simulink model "vegas01_4tap_1024ch_250mhz_r1"  (Simulink 7.6)
settings: Solver=ode45  StartTime=0.0  StopTime=128000  RelTol=1e-3
undefined symbols (not defined in the file): ROACH
libraries: xbsIndex_r4 x12350, monroe_library x84, xbsReplacements_r4 x36, xps_library x30, casper_library_misc x24, dspsigops x16
(part 7 of 103)

== system vegas01_4tap_1024ch_250mhz_r1/pfb_fir_real1/coeff_gen_3_4 ==
  sync : Inport
  Counter0 : xbsIndex_r4/Counter  cnt_type=Free Running  cnt_to=Inf  operation=Up  start_count=0  cnt_by_val=1  arith_type=Unsigned  n_bits=8  bin_pt=0  load_pin=off  rst=on  en=off  explicit_period=on  period=1  dbl_ovrd=off  use_behavioral_HDL=off  implementation=Fabric  block_type=counter
  Counter1 : xbsIndex_r4/Counter  cnt_type=Free Running  cnt_to=Inf  operation=Down  start_count=255  cnt_by_val=1  arith_type=Unsigned  n_bits=8  bin_pt=0  load_pin=off  rst=on  en=off  explicit_period=on  period=1  dbl_ovrd=off  use_behavioral_HDL=off  implementation=Fabric  block_type=counter
  coeff_ram_1 : xbsIndex_r4/Dual Port RAM  depth=256  initVector=[-5.86373825914179e-05 -0.000215456147790523 -0.000372998021380911 -0.000531345655026345 -0.000690582222902466 -0.000850791372601726 -0.00101205717553319 -0.00117446407683278 -0.00133809684480029 -0.0015030405198794 -0.00166938036319721 -0.00183720180467995 -0.00200659039076204 -0.0021776317317052 -0.00235041144854523 -0.00252501511968397 -0.00270152822714364 -0.00288003610250207 -0.00306062387252614 -0.00324337640452213 -0.00342837825142082 -0.00361571359661625 -0.00380546619857627 -0.00399771933524413 -0.00419255574824945 -0.00439005758694802 -0.00459030635230934 -0.00479338284067104 -0.00499936708737985 -0.00520833831033822 -0.00542037485347644 -0.00563555413016958 -0.00585395256661942 -0.0060756455452207 -0.00630070734793194 -0.00652921109967067 -0.00676122871175293 -0.00699683082539746 -0.00723608675531428 -0.00747906443339831 -0.00772583035254768 -0.00797644951062753 -0.00823098535459909 -0.00848949972483472 -0.00875205279963877 -0.00901870303999501 -0.00928950713456065 -0.00956451994492721 -0.0098437944511688 -0.0101273816976976 -0.0104153307394476 -0.0107076885884055 -0.0110045001605102 -0.0113058082229405 -0.0116116533418109 -0.0119220738302957 -0.0122371056972012 -0.0125567825960062 -0.0128811357743903 -0.0132101940242697 -0.0135439836323603 -0.0138825283312874 -0.0142258492512617 -0.0145739648723409 -0.0149268909772952 -0.0152846406050976 -0.0156472240050563 -0.0160146485916082 -0.0163869188997935 -0.0167640365414281 -0.0171460001619934 -0.0175328053982617 -0.0179244448366743 -0.0183209079724907 -0.0187221811697278 -0.0191282476219039 -0.0195390873136075 -0.0199546769829069 -0.0203749900846166 -0.0207999967544396 -0.0212296637739995 -0.0216639545367806 -0.0221028290149906 -0.0225462437273637 -0.0229941517079172 -0.0234465024756791 -0.0239032420054004 -0.0243643126992679 -0.0248296533596316 -0.0252991991627612 -0.0257728816336456 -0.0262506286218495 -0.0267323642784407 -0.0272180090340002 -0.0277074795777305 -0.0282006888376717 -0.0286975459620406 -0.0291979563017022 -0.0297018213937885 -0.0302090389464727 -0.0307195028249126 -0.0312331030383722 -0.0317497257285335 -0.0322692531590073 -0.0327915637060538 -0.0333165318505221 -0.033844028171018 -0.0343739193383085 -0.0349060681109713 -0.0354403333322995 -0.0359765699284654 -0.0365146289079547 -0.0370543573622759 -0.0375955984679522 -0.0381381914898021 -0.038681971785515 -0.0392267708115271 -0.0397724161302025 -0.0403187314183254 -0.0408655364769064 -0.0414126472423082 -0.0419598757986934 -0.0425070303917987 -0.0430539154440375 -0.043600331570934 -0.0441460755988906 -0.0446909405842904 -0.0452347158339363 -0.0457771869268272 -0.0463181357372728 -0.0468573404593456 -0.047394575632671 -0.047929612169555 -0.0484622173834472 -0.0489921550187384 -0.0495191852818918 -0.0500430648739029 -0.0505635470240889 -0.0510803815252008 -0.051593314769858 -0.0521020897882994 -0.0526064462874469 -0.0531061206912774 -0.0536008461824974 -0.0540903527455154 -0.0545743672107048 -0.0550526132999523 -0.0555248116734847 -0.0559906799779658 -0.056449932895858 -0.0569022821960385 -0.0573474367856626 -0.0577851027632654 -0.058214983473093 -0.0586367795606527 -0.0590501890294728 -0.0594549072990618 -0.0598506272640558 -0.0602370393545433 -0.0606138315975561 -0.0609806896797129 -0.0613372970110058 -0.0616833347897144 -0.0620184820684369 -0.0623424158212223 -0.0626548110117918 -0.062955340662835 -0.0632436759263647 -0.0635194861551178 -0.0637824389749851 -0.0640322003584556 -0.0642684346990587 -0.064490804886788 -0.0646989723844908 -0.0648925973052045 -0.0650713384904255 -0.0652348535892895 -0.0653827991386482 -0.0655148306440217 -0.0656306026614101 -0.0657297688799434 -0.0658119822053517 -0.0658768948442361 -0.0659241583891191 -0.0659534239042566 -0.0659643420121883 -0.0659565629810087 -0.065929736812335 -0.0658835133299523 -0.0658175422691142 -0.0657314733664758 -0.0656249564506396 -0.065497641533289 -0.0653491789008882 -0.0651792192069268 -0.0649874135646831 -0.0647734136404861 -0.0645368717474501 ...(+1155 chars)]  distributed_mem=Block RAM  init_a=0  init_b=0  rst_a=off  rst_b=off  en_a=off  en_b=off  latency=3  write_mode_A=No Read On Write  write_mode_B=No Read On Write  dbl_ovrd=off  optimize=Area  use_rpm=on  block_type=dpram
  coeff_ram_2 : xbsIndex_r4/Dual Port RAM  depth=256  initVector=[0.000793855568781102 0.00293734991211368 0.00511955769539444 0.00734060096668454 0.00960059539061112 0.0118996501782322 0.0142378680181029 0.016615345008565 0.0190321705912827 0.0214884274860464 0.0239841916268662 0.0265195320993763 0.0290945110795724 0.0317091837739016 0.0343635983607266 0.0370577959331841 0.0397918104434568 0.04256566864848 0.0453793900570998 0.0482329868787049 0.051126463973348 0.0540598188033769 0.0570330413865915 0.0600461142509466 0.0630990123908156 0.066191703224833 0.0693241465553323 0.072496294529395 0.0757080916015266 0.0789594744979745 0.0822503721827049 0.0855807058250493 0.0889503887690387 0.0923593265044371 0.095807416639488 0.0992945488753877 0.102820604982496 0.1063854587783 0.109988976107135 0.113631014821687 0.117311424766273 0.121030047761919 0.124786717593239 0.128581259997137 0.132413492653321 0.136283225176654 0.140190259111347 0.144134387926999 0.148115397016481 0.152133063695701 0.156187157205215 0.16027743871373 0.164403661323472 0.168565570077445 0.172762901968574 0.176995385950742 0.18126274295172 0.185564685887996 0.189900919681506 0.19427114127826 0.198675039668881 0.203112295911043 0.207582583153816 0.21208556666391 0.216620903853836 0.221188244311956 0.225787229834444 0.230417494459145 0.235078664501327 0.239770358591334 0.244492187714121 0.249243755250686 0.254024657021376 0.258834481331072 0.263672809016247 0.268539213493888 0.273433260812281 0.278354509703641 0.283302511638591 0.28827681088248 0.293276944553524 0.29830244268277 0.303352828275865 0.308427617376632 0.31352631913243 0.318648435861293 0.323793463120839 0.328960889778932 0.334150198086085 0.339360863749602 0.344592356009424 0.349844137715687 0.355115665407971 0.360406389396214 0.365715753843293 0.371043196849245 0.376388150537122 0.381750041140444 0.387128289092261 0.392522309115784 0.397931510316576 0.403355296276285 0.408793065147898 0.414244209752503 0.419708117677526 0.42518417137644 0.430671748269917 0.436170220848394 0.441678956776052 0.447197318996171 0.452724665837839 0.458260351124004 0.463803724280834 0.469354130448373 0.474910910592456 0.480473401617876 0.486040936482762 0.491612844314159 0.497188450524771 0.502767076930856 0.508348041871229 0.513930660327374 0.519514244044604 0.525098101654283 0.530681538797043 0.536263858246998 0.541844360036917 0.547422341584325 0.552997097818512 0.558567921308413 0.564134102391344 0.569694929302543 0.575249688305515 0.580797663823129 0.586338138569444 0.591870393682245 0.597393708856244 0.602907362476923 0.608410631754986 0.613902792861399 0.61938312106297 0.624850890858456 0.630305376115151 0.635745850205934 0.641171586146744 0.646581856734442 0.651975934685042 0.657353092772267 0.662712603966406 0.668053741573435 0.673375779374372 0.678677991764833 0.683959653894763 0.689220041808292 0.694458432583704 0.699674104473478 0.704866337044359 0.710034411317448 0.715177609908251 0.72029521716668 0.725386519316956 0.730450804597387 0.735487363399991 0.740495488409925 0.745474474744689 0.750423620093078 0.755342224853842 0.760229592274024 0.76508502858694 0.769907843149779 0.774697348580773 0.779452860895924 0.784173699645239 0.788859188048447 0.793508653130172 0.79812142585452 0.802696841259047 0.807234238588094 0.811732961425428 0.816192357826184 0.820611780448065 0.824990586681763 0.829328138780581 0.83362380398922 0.837876954671699 0.842086968438377 0.846253228272049 0.850375122653088 0.854452045683591 0.858483397210515 0.862468582947766 0.866407014597203 0.870298109968547 0.874141293098149 0.877935994366595 0.88168165061512 0.885377705260805 0.889023608410519 0.892618816973592 0.896162794773185 0.899655012656325 0.90309494860259 0.906482087831407 0.909815922907943 0.913095953847561 0.916321688218814 0.919492641244963 0.922608335903972 0.925668303026978 0.928672081395204 0.931619217835282 0.934509267312983 0.937341793025309 0.940116366490941 0.942832567639016 0.945489984896204 0.948088215272075 0.950626864442732 0.953105546832681 0.955523885694932 0.9578815131893 ...(+626 chars)]  distributed_mem=Block RAM  init_a=0  init_b=0  rst_a=off  rst_b=off  en_a=off  en_b=off  latency=3  write_mode_A=No Read On Write  write_mode_B=No Read On Write  dbl_ovrd=off  optimize=Area  use_rpm=on  block_type=dpram
  coeff_ram_3 : xbsIndex_r4/Dual Port RAM  depth=256  initVector=[0.999994812497531 0.999934649315113 0.999806974692208 0.999611808340819 0.999349180372279 0.999019131290932 0.998621711985642 0.998156983719109 0.99762501811503 0.997025897143069 0.996359713101675 0.995626568598733 0.994826576530044 0.993959860055675 0.993026552574138 0.992026797694442 0.990960749206007 0.989828571046454 0.988630437267265 0.987366531997347 0.986037049404487 0.984642193654713 0.983182178869577 0.981657229081366 0.980067578186247 0.978413469895374 0.976695157683941 0.974912904738229 0.973066983900625 0.971157677612657 0.96918527785603 0.967150086091704 0.965052413197015 0.962892579400849 0.9606709142169 0.95838775637502 0.956043453750677 0.953638363292534 0.951172850948186 0.948647291588047 0.946062068927424 0.94341757544679 0.940714212310279 0.937952389282417 0.935132524643115 0.932255045100944 0.929320385704707 0.926328989753344 0.923281308704171 0.920177802079496 0.917018937371619 0.913805189946252 0.910537042944371 0.907214987182531 0.903839521051669 0.90041115041441 0.896930388500917 0.893397755803297 0.889813779968586 0.886178995690362 0.88249394459898 0.878759175150481 0.87497524251419 0.871142708459034 0.867262141238604 0.863334115474993 0.85935921204144 0.855338017943796 0.851271126200856 0.847159135723579 0.843002651193223 0.83880228293843 0.834558646811279 0.83027236406236 0.825944061214867 0.821574369937774 0.817163926918096 0.81271337373228 0.808223356716763 0.803694526837708 0.799127539559968 0.7945230547153 0.789881736369862 0.785204252691029 0.780491275813551 0.775743481705095 0.770961550031191 0.766146164019636 0.761298010324352 0.756417778888776 0.751506162808768 0.74656385819511 0.741591564035602 0.736589982056795 0.731559816585398 0.726501774409391 0.721416564638871 0.716304898566669 0.711167489528765 0.706005052764548 0.700818305276932 0.695607965692387 0.690374754120884 0.685119392015829 0.679842602033979 0.674545107895397 0.669227634243474 0.663890906505042 0.658535650750622 0.653162593554832 0.647772461856991 0.642365982821949 0.636943883701178 0.631506891694151 0.626055733810047 0.620591136729813 0.61511382666861 0.609624529238677 0.604123969312646 0.598612870887343 0.593091956948089 0.587561949333563 0.582023568601217 0.576477533893316 0.570924562803598 0.56536537124461 0.559800673315729 0.554231181171917 0.548657604893226 0.543080652355085 0.537501029099403 0.53191943820651 0.526336580167975 0.520753152760311 0.515169850919615 0.50958736661716 0.504006388735964 0.498427602948374 0.492851691594681 0.487279333562795 0.481711204169014 0.476147975039901 0.470590313995303 0.465038884932535 0.459494347711752 0.453957358042535 0.448428567371718 0.442908622772472 0.437398166834681 0.431897837556619 0.426408268237969 0.420930087374181 0.415463918552222 0.410010380347709 0.404570086223473 0.39914364442955 0.393731657904643 0.388334724179058 0.382953435279135 0.377588377633211 0.372240131979102 0.366909273273159 0.361596370600881 0.35630198708913 0.351026679819948 0.345770999746003 0.340535491607668 0.335320693851764 0.330127138551971 0.324955351330922 0.319805851284001 0.314679150904859 0.309575756012646 0.304496165680994 0.299440872168748 0.294410360852464 0.28940511016068 0.284425591509976 0.279472269242834 0.274545600567304 0.269646035498482 0.264774016801823 0.259929979938285 0.255114353011316 0.250327556715688 0.245570004288195 0.240842101460215 0.236144246412134 0.231476829729653 0.226840234361979 0.222234835581892 0.21766100094771 0.213119090267143 0.208609455563046 0.20413244104107 0.199688383059216 0.195277610099287 0.19090044274025 0.186557193633502 0.182248167480034 0.177973661009513 0.173733962961252 0.169529354067092 0.165360107036185 0.161226486541674 0.15712874920926 0.153067143607678 0.149041910241047 0.14505328154311 0.141101481873355 0.137186727515006 0.133309226674887 0.129469179485146 0.125666778006837 0.121902206235346 0.118175640107666 0.114487247511497 0.110837188296181 0.107225614285441 0.103652669291933 0.100118489133601 0.0966232016518021 0.0931669267312255 ...(+627 chars)]  distributed_mem=Block RAM  init_a=0  init_b=0  rst_a=off  rst_b=off  en_a=off  en_b=off  latency=3  write_mode_A=No Read On Write  write_mode_B=No Read On Write  dbl_ovrd=off  optimize=Area  use_rpm=on  block_type=dpram
  coeff_ram_4 : xbsIndex_r4/Dual Port RAM  depth=256  initVector=[-0.000787920351828344 -0.00286260996943633 -0.00489896520129326 -0.00689713161888919 -0.00885726082449819 -0.0107795103745431 -0.0126640437019187 -0.0145110300372983 -0.0163206443294465 -0.0180930671645657 -0.0198284846846987 -0.0215270885052133 -0.0231890756313952 -0.0248146483741729 -0.0264040142650019 -0.0279573859699323 -0.0294749812028871 -0.0309570226381763 -0.0324037378222731 -0.0338153590848793 -0.0351921234493043 -0.036534272542187 -0.0378420525025839 -0.0391157138904531 -0.0403555115945585 -0.0415617047398231 -0.042734556594156 -0.0438743344747823 -0.0449813096541012 -0.0460557572651 -0.0470979562063509 -0.0481081890466181 -0.0490867419291014 -0.0500339044753439 -0.0509499696888316 -0.0518352338583101 -0.0526899964608479 -0.0535145600646716 -0.0543092302318 -0.0550743154205058 -0.0558101268876299 -0.0565169785907762 -0.0571951870904141 -0.0578450714519141 -0.0584669531475448 -0.0590611559584568 -0.0596280058766808 -0.0601678310071659 -0.0606809614698843 -0.0611677293020293 -0.0616284683603321 -0.0620635142235239 -0.0624732040949686 -0.0628578767054923 -0.0632178722164352 -0.0635535321229509 -0.063865199157579 -0.0641532171941154 -0.0644179311518061 -0.0646596868998882 -0.0648788311625045 -0.0650757114240131 -0.0652506758347198 -0.0654040731170541 -0.065536252472215 -0.0656475634873088 -0.0657383560430019 -0.0658089802217137 -0.0658597862163704 -0.0658911242397428 -0.0659033444343921 -0.0658967967832431 -0.0658718310208095 -0.0658287965450905 -0.0657680423301619 -0.0656899168394816 -0.0655947679399311 -0.0654829428166129 -0.0653547878884241 -0.0652106487244269 -0.0650508699610347 -0.0648757952200333 -0.0646857670274563 -0.0644811267333347 -0.0642622144323359 -0.0640293688853139 -0.0637829274417857 -0.0635232259633528 -0.0632505987480838 -0.0629653784558762 -0.0626678960348121 -0.0623584806485253 -0.0620374596045948 -0.0617051582839797 -0.0613619000715118 -0.0610080062874585 -0.0606437961201723 -0.0602695865598394 -0.0598856923333414 -0.0594924258402432 -0.0590900970899198 -0.0586790136398347 -0.0582594805349814 -0.0578318002484996 -0.0573962726234782 -0.0569531948159544 -0.0565028612391215 -0.0560455635087529 -0.0555815903898541 -0.0551112277445508 -0.0546347584812225 -0.0541524625048896 -0.0536646166688625 -0.0531714947276601 -0.0526733672912054 -0.0521705017803039 -0.051663162383413 -0.051151610014707 -0.0506361022734436 -0.0501168934046382 -0.0495942342610489 -0.0490683722664788 -0.0485395513803979 -0.0480080120638888 -0.0474739912469198 -0.0469377222969473 -0.0463994349888512 -0.0458593554762035 -0.0453177062638732 -0.0447747061819681 -0.0442305703611143 -0.0436855102090731 -0.0431397333886967 -0.0425934437972209 -0.0420468415468947 -0.0415001229469451 -0.0409534804868759 -0.040407102821098 -0.0398611747548894 -0.0393158772316811 -0.0387713873216666 -0.0382278782117312 -0.0376855191966968 -0.0371444756718779 -0.0366049091269439 -0.0360669771410839 -0.035530833379466 -0.0349966275909875 -0.0344645056073088 -0.0339346093431641 -0.0334070767979427 -0.0328820420585328 -0.0323596353034207 -0.0318399828080369 -0.0313232069513411 -0.0308094262236367 -0.0302987552356068 -0.0297913047285612 -0.0292871815858849 -0.0287864888456782 -0.0282893257145784 -0.0277957875827514 -0.0273059660400422 -0.0268199488932742 -0.0263378201846839 -0.0258596602114802 -0.0253855455465156 -0.024915549060056 -0.0244497399426378 -0.0239881837289974 -0.0235309423230611 -0.0230780740239801 -0.0226296335531983 -0.0221856720825369 -0.0217462372632829 -0.0213113732562652 -0.0208811207629043 -0.0204555170572198 -0.02003459601878 -0.0196183881665788 -0.019206920693822 -0.0188002175036087 -0.0183982992454906 -0.0180011833528919 -0.0176088840813737 -0.0172214125477258 -0.0168387767698682 -0.0164609817075447 -0.0160880293037919 -0.0157199185271648 -0.015356645414701 -0.0149982031156059 -0.0146445819356409 -0.0142957693821939 -0.013951750210017 -0.0136125064676089 -0.0132780175442264 -0.012948260217503 -0.012623208701658 -0.0123028346962748 ...(+1170 chars)]  distributed_mem=Block RAM  init_a=0  init_b=0  rst_a=off  rst_b=off  en_a=off  en_b=off  latency=3  write_mode_A=No Read On Write  write_mode_B=No Read On Write  dbl_ovrd=off  optimize=Area  use_rpm=on  block_type=dpram
  const_din : xbsIndex_r4/Constant  arith_type=Signed (2's comp)  const=0  n_bits=18  bin_pt=17  explicit_period=on  period=1  equ=P=C  opselect=C  inp2=PCIN>>17  opr=+  inp1=P  carry=CIN  dbl_ovrd=off  block_type=constant
  const_we : xbsIndex_r4/Constant  arith_type=Boolean  const=0  n_bits=16  bin_pt=14  explicit_period=on  period=1  equ=P=C  opselect=C  inp2=PCIN>>17  opr=+  inp1=P  carry=CIN  dbl_ovrd=off  block_type=constant
  cram_lower : Subsystem
  cram_upper : Subsystem
  delay_a_addr1 : xbsIndex_r4/Delay  en=off  latency=2  dbl_ovrd=off  reg_retiming=off  block_type=delay
  delay_a_addr2 : xbsIndex_r4/Delay  en=off  latency=1  dbl_ovrd=off  reg_retiming=off  block_type=delay
  delay_b_addr3 : xbsIndex_r4/Delay  en=off  latency=1  dbl_ovrd=off  reg_retiming=off  block_type=delay
  delay_b_addr4 : xbsIndex_r4/Delay  en=off  latency=2  dbl_ovrd=off  reg_retiming=off  block_type=delay
  delay_sync : xbsIndex_r4/Delay  en=off  latency=3  dbl_ovrd=off  reg_retiming=off  block_type=delay
  sync_out : Outport
  coeff : Outport  Port=2
  coeff_rev : Outport  Port=3
  wires:
  sync/1 -> Counter1/1, Counter0/1, delay_sync/1
  Counter0/1 -> coeff_ram_4/1, coeff_ram_3/1, delay_a_addr2/1, delay_a_addr1/1
  const_din/1 -> coeff_ram_4/5, coeff_ram_4/2, coeff_ram_3/5, coeff_ram_3/2, coeff_ram_2/5, coeff_ram_2/2, coeff_ram_1/5, coeff_ram_1/2
  const_we/1 -> coeff_ram_4/6, coeff_ram_4/3, coeff_ram_3/6, coeff_ram_3/3, coeff_ram_2/6, coeff_ram_2/3, coeff_ram_1/6, coeff_ram_1/3
  Counter1/1 -> delay_b_addr4/1, delay_b_addr3/1, coeff_ram_2/4, coeff_ram_1/4
  cram_lower/1(out) -> coeff_rev/1
  cram_upper/1(out) -> coeff/1
  coeff_ram_1/2 -> cram_lower/4(in3)
  coeff_ram_2/2 -> cram_lower/3(in2)
  coeff_ram_3/2 -> cram_lower/2(in1)
  coeff_ram_4/2 -> cram_lower/1(in0)
  coeff_ram_4/1 -> cram_upper/4(in3)
  coeff_ram_3/1 -> cram_upper/3(in2)
  coeff_ram_2/1 -> cram_upper/2(in1)
  coeff_ram_1/1 -> cram_upper/1(in0)
  delay_b_addr4/1 -> coeff_ram_4/4
  delay_b_addr3/1 -> coeff_ram_3/4
  delay_a_addr2/1 -> coeff_ram_2/1
  delay_a_addr1/1 -> coeff_ram_1/1
  delay_sync/1 -> sync_out/1

== system vegas01_4tap_1024ch_250mhz_r1/pfb_fir_real1/multi_delay_bram_fast_0 ==
  in0 : Inport
  in1 : Inport  Port=2
  in2 : Inport  Port=3
  in3 : Inport  Port=4
  in4 : Inport  Port=5
  in5 : Inport  Port=6
  Concat2_1 : xbsIndex_r4/Concat  num_inputs=2  dbl_ovrd=off  block_type=concat
  cram : Subsystem
  cram_all : Subsystem
  double_delay_bram_fast1 : monroe_library/double_delay_bram_fast  SystemSampleTime=-1  delay_len=256  bram_latency=2  register_counter=1
  double_delay_bram_fast2 : monroe_library/delay_bram_fast  SystemSampleTime=-1  delay_len=256  bram_latency=2  memory_type=Block RAM  register_counter=1
  sliceA_1 : xbsIndex_r4/Slice  nbits=18  boolean_output=off  mode=Upper Bit Location + Width  bit1=-0  base1=MSB of Input  bit0=-0  base0=MSB of Input  dbl_ovrd=off  block_type=slice
  sliceB_1 : xbsIndex_r4/Slice  nbits=18  boolean_output=off  mode=Upper Bit Location + Width  bit1=-18  base1=MSB of Input  bit0=-18  base0=MSB of Input  dbl_ovrd=off  block_type=slice
  slice_last : xbsIndex_r4/Slice  nbits=12  boolean_output=off  mode=Lower Bit Location + Width  bit1=0  base1=LSB of Input  bit0=0  base0=LSB of Input  dbl_ovrd=off  block_type=slice
  uncram : Subsystem
  out0 : Outport
  out1 : Outport  Port=2
  out2 : Outport  Port=3
  out3 : Outport  Port=4
  out4 : Outport  Port=5
  out5 : Outport  Port=6
  wires:
  cram/1(out) -> slice_last/1, sliceB_1/1, sliceA_1/1
  uncram/6(out5) -> out5/1
  uncram/5(out4) -> out4/1
  uncram/4(out3) -> out3/1
  uncram/3(out2) -> out2/1
  uncram/2(out1) -> out1/1
  uncram/1(out0) -> out0/1
  cram_all/1(out) -> uncram/1(in)
  double_delay_bram_fast2/1 -> cram_all/2(in1)
  Concat2_1/1 -> cram_all/1(in0)
  slice_last/1 -> double_delay_bram_fast2/1
  double_delay_bram_fast1/2 -> Concat2_1/2
  double_delay_bram_fast1/1 -> Concat2_1/1
  sliceB_1/1 -> double_delay_bram_fast1/2
  sliceA_1/1 -> double_delay_bram_fast1/1
  in5/1 -> cram/6(in5)
  in4/1 -> cram/5(in4)
  in3/1 -> cram/4(in3)
  in2/1 -> cram/3(in2)
  in1/1 -> cram/2(in1)
  in0/1 -> cram/1(in0)

== system vegas01_4tap_1024ch_250mhz_r1/pfb_fir_real1/multi_delay_bram_fast_1 ==
  in0 : Inport
  in1 : Inport  Port=2
  in2 : Inport  Port=3
  in3 : Inport  Port=4
  in4 : Inport  Port=5
  in5 : Inport  Port=6
  Concat2_1 : xbsIndex_r4/Concat  num_inputs=2  dbl_ovrd=off  block_type=concat
  cram : Subsystem
  cram_all : Subsystem
  double_delay_bram_fast1 : monroe_library/double_delay_bram_fast  SystemSampleTime=-1  delay_len=256  bram_latency=2  register_counter=1
  double_delay_bram_fast2 : monroe_library/delay_bram_fast  SystemSampleTime=-1  delay_len=256  bram_latency=2  memory_type=Block RAM  register_counter=1
  sliceA_1 : xbsIndex_r4/Slice  nbits=18  boolean_output=off  mode=Upper Bit Location + Width  bit1=-0  base1=MSB of Input  bit0=-0  base0=MSB of Input  dbl_ovrd=off  block_type=slice
  sliceB_1 : xbsIndex_r4/Slice  nbits=18  boolean_output=off  mode=Upper Bit Location + Width  bit1=-18  base1=MSB of Input  bit0=-18  base0=MSB of Input  dbl_ovrd=off  block_type=slice
  slice_last : xbsIndex_r4/Slice  nbits=12  boolean_output=off  mode=Lower Bit Location + Width  bit1=0  base1=LSB of Input  bit0=0  base0=LSB of Input  dbl_ovrd=off  block_type=slice
  uncram : Subsystem
  out0 : Outport
  out1 : Outport  Port=2
  out2 : Outport  Port=3
  out3 : Outport  Port=4
  out4 : Outport  Port=5
  out5 : Outport  Port=6
  wires:
  cram/1(out) -> slice_last/1, sliceB_1/1, sliceA_1/1
  uncram/6(out5) -> out5/1
  uncram/5(out4) -> out4/1
  uncram/4(out3) -> out3/1
  uncram/3(out2) -> out2/1
  uncram/2(out1) -> out1/1
  uncram/1(out0) -> out0/1
  cram_all/1(out) -> uncram/1(in)
  double_delay_bram_fast2/1 -> cram_all/2(in1)
  Concat2_1/1 -> cram_all/1(in0)
  slice_last/1 -> double_delay_bram_fast2/1
  double_delay_bram_fast1/2 -> Concat2_1/2
  double_delay_bram_fast1/1 -> Concat2_1/1
  sliceB_1/1 -> double_delay_bram_fast1/2
  sliceA_1/1 -> double_delay_bram_fast1/1
  in5/1 -> cram/6(in5)
  in4/1 -> cram/5(in4)
  in3/1 -> cram/4(in3)
  in2/1 -> cram/3(in2)
  in1/1 -> cram/2(in1)
  in0/1 -> cram/1(in0)

== system vegas01_4tap_1024ch_250mhz_r1/pfb_fir_real1/multi_delay_bram_fast_2 ==
  in0 : Inport
  in1 : Inport  Port=2
  in2 : Inport  Port=3
  in3 : Inport  Port=4
  in4 : Inport  Port=5
  in5 : Inport  Port=6
  Concat2_1 : xbsIndex_r4/Concat  num_inputs=2  dbl_ovrd=off  block_type=concat
  cram : Subsystem
  cram_all : Subsystem
  double_delay_bram_fast1 : monroe_library/double_delay_bram_fast  SystemSampleTime=-1  delay_len=256  bram_latency=2  register_counter=1
  double_delay_bram_fast2 : monroe_library/delay_bram_fast  SystemSampleTime=-1  delay_len=256  bram_latency=2  memory_type=Block RAM  register_counter=1
  sliceA_1 : xbsIndex_r4/Slice  nbits=18  boolean_output=off  mode=Upper Bit Location + Width  bit1=-0  base1=MSB of Input  bit0=-0  base0=MSB of Input  dbl_ovrd=off  block_type=slice
  sliceB_1 : xbsIndex_r4/Slice  nbits=18  boolean_output=off  mode=Upper Bit Location + Width  bit1=-18  base1=MSB of Input  bit0=-18  base0=MSB of Input  dbl_ovrd=off  block_type=slice
  slice_last : xbsIndex_r4/Slice  nbits=12  boolean_output=off  mode=Lower Bit Location + Width  bit1=0  base1=LSB of Input  bit0=0  base0=LSB of Input  dbl_ovrd=off  block_type=slice
  uncram : Subsystem
  out0 : Outport
  out1 : Outport  Port=2
  out2 : Outport  Port=3
  out3 : Outport  Port=4
  out4 : Outport  Port=5
  out5 : Outport  Port=6
  wires:
  cram/1(out) -> slice_last/1, sliceB_1/1, sliceA_1/1
  uncram/6(out5) -> out5/1
  uncram/5(out4) -> out4/1
  uncram/4(out3) -> out3/1
  uncram/3(out2) -> out2/1
  uncram/2(out1) -> out1/1
  uncram/1(out0) -> out0/1
  cram_all/1(out) -> uncram/1(in)
  double_delay_bram_fast2/1 -> cram_all/2(in1)
  Concat2_1/1 -> cram_all/1(in0)
  slice_last/1 -> double_delay_bram_fast2/1
  double_delay_bram_fast1/2 -> Concat2_1/2
  double_delay_bram_fast1/1 -> Concat2_1/1
  sliceB_1/1 -> double_delay_bram_fast1/2
  sliceA_1/1 -> double_delay_bram_fast1/1
  in5/1 -> cram/6(in5)
  in4/1 -> cram/5(in4)
  in3/1 -> cram/4(in3)
  in2/1 -> cram/3(in2)
  in1/1 -> cram/2(in1)
  in0/1 -> cram/1(in0)

== system vegas01_4tap_1024ch_250mhz_r1/pfb_fir_real1/multi_delay_bram_fast_3 ==
  in0 : Inport
  in1 : Inport  Port=2
  in2 : Inport  Port=3
  in3 : Inport  Port=4
  in4 : Inport  Port=5
  in5 : Inport  Port=6
  Concat2_1 : xbsIndex_r4/Concat  num_inputs=2  dbl_ovrd=off  block_type=concat
  cram : Subsystem
  cram_all : Subsystem
  double_delay_bram_fast1 : monroe_library/double_delay_bram_fast  SystemSampleTime=-1  delay_len=256  bram_latency=2  register_counter=1
  double_delay_bram_fast2 : monroe_library/delay_bram_fast  SystemSampleTime=-1  delay_len=256  bram_latency=2  memory_type=Block RAM  register_counter=1
  sliceA_1 : xbsIndex_r4/Slice  nbits=18  boolean_output=off  mode=Upper Bit Location + Width  bit1=-0  base1=MSB of Input  bit0=-0  base0=MSB of Input  dbl_ovrd=off  block_type=slice
  sliceB_1 : xbsIndex_r4/Slice  nbits=18  boolean_output=off  mode=Upper Bit Location + Width  bit1=-18  base1=MSB of Input  bit0=-18  base0=MSB of Input  dbl_ovrd=off  block_type=slice
  slice_last : xbsIndex_r4/Slice  nbits=12  boolean_output=off  mode=Lower Bit Location + Width  bit1=0  base1=LSB of Input  bit0=0  base0=LSB of Input  dbl_ovrd=off  block_type=slice
  uncram : Subsystem
  out0 : Outport
  out1 : Outport  Port=2
  out2 : Outport  Port=3
  out3 : Outport  Port=4
  out4 : Outport  Port=5
  out5 : Outport  Port=6
  wires:
  cram/1(out) -> slice_last/1, sliceB_1/1, sliceA_1/1
  uncram/6(out5) -> out5/1
  uncram/5(out4) -> out4/1
  uncram/4(out3) -> out3/1
  uncram/3(out2) -> out2/1
  uncram/2(out1) -> out1/1
  uncram/1(out0) -> out0/1
  cram_all/1(out) -> uncram/1(in)
  double_delay_bram_fast2/1 -> cram_all/2(in1)
  Concat2_1/1 -> cram_all/1(in0)
  slice_last/1 -> double_delay_bram_fast2/1
  double_delay_bram_fast1/2 -> Concat2_1/2
  double_delay_bram_fast1/1 -> Concat2_1/1
  sliceB_1/1 -> double_delay_bram_fast1/2
  sliceA_1/1 -> double_delay_bram_fast1/1
  in5/1 -> cram/6(in5)
  in4/1 -> cram/5(in4)
  in3/1 -> cram/4(in3)
  in2/1 -> cram/3(in2)
  in1/1 -> cram/2(in1)
  in0/1 -> cram/1(in0)
